simulink library "Garbage"  (R2023a)
settings: Solver=ode45  StartTime=0.0  StopTime=10.0  RelTol=1e-3  EnableMultiTasking=on
undefined symbols (not defined in the file or in its folder): c, ending_column_index, ending_row_index, framebuffer_16x1, framebuffer_2x2, framebuffer_4x4, framebuffer_8x8, HEIGHT, hot, POS_X, POS_Y, starting_column_index, starting_row_index, WIDTH
libraries: Memory_Blocks_Lib x459, simulink x235, simulink_extras x132, dspmtrx3 x116, Bit_Multiplexers_Lib x67, Bit_Demultiplexers_Lib x49, Byte_Multiplexers_Lib x40, Miscellaneous x38, Computer_Library x17, Decoders_Lib x14, commutil2 x12, Arithmetic_Operations_Lib x8
(part 10 of 26)

== system Garbage/Encoder_128_to_7 ==
  Select_127(1b) : Inport
  Select_126(1b) : Inport  Port=2
  Select_125(1b) : Inport  Port=3
  Select_124(1b) : Inport  Port=4
  Select_123(1b) : Inport  Port=5
  Select_122(1b) : Inport  Port=6
  Select_121(1b) : Inport  Port=7
  Select_120(1b) : Inport  Port=8
  Select_119(1b) : Inport  Port=9
  Select_118(1b) : Inport  Port=10
  Select_117(1b) : Inport  Port=11
  Select_116(1b) : Inport  Port=12
  Select_115(1b) : Inport  Port=13
  Select_114(1b) : Inport  Port=14
  Select_113(1b) : Inport  Port=15
  Select_112(1b) : Inport  Port=16
  Select_111(1b) : Inport  Port=17
  Select_110(1b) : Inport  Port=18
  Select_109(1b) : Inport  Port=19
  Select_108(1b) : Inport  Port=20
  Select_107(1b) : Inport  Port=21
  Select_106(1b) : Inport  Port=22
  Select_105(1b) : Inport  Port=23
  Select_104(1b) : Inport  Port=24
  Select_103(1b) : Inport  Port=25
  Select_102(1b) : Inport  Port=26
  Select_101(1b) : Inport  Port=27
  Select_100(1b) : Inport  Port=28
  Select_099(1b) : Inport  Port=29
  Select_098(1b) : Inport  Port=30
  Select_097(1b) : Inport  Port=31
  Select_096(1b) : Inport  Port=32
  Select_095(1b) : Inport  Port=33
  Select_094(1b) : Inport  Port=34
  Select_093(1b) : Inport  Port=35
  Select_092(1b) : Inport  Port=36
  Select_091(1b) : Inport  Port=37
  Select_090(1b) : Inport  Port=38
  Select_089(1b) : Inport  Port=39
  Select_088(1b) : Inport  Port=40
  Select_087(1b) : Inport  Port=41
  Select_086(1b) : Inport  Port=42
  Select_085(1b) : Inport  Port=43
  Select_084(1b) : Inport  Port=44
  Select_083(1b) : Inport  Port=45
  Select_082(1b) : Inport  Port=46
  Select_081(1b) : Inport  Port=47
  Select_080(1b) : Inport  Port=48
  Select_079(1b) : Inport  Port=49
  Select_078(1b) : Inport  Port=50
  Select_077(1b) : Inport  Port=51
  Select_076(1b) : Inport  Port=52
  Select_075(1b) : Inport  Port=53
  Select_074(1b) : Inport  Port=54
  Select_073(1b) : Inport  Port=55
  Select_072(1b) : Inport  Port=56
  Select_071(1b) : Inport  Port=57
  Select_070(1b) : Inport  Port=58
  Select_069(1b) : Inport  Port=59
  Select_068(1b) : Inport  Port=60
  Select_067(1b) : Inport  Port=61
  Select_066(1b) : Inport  Port=62
  Select_065(1b) : Inport  Port=63
  Select_064(1b) : Inport  Port=64
  Select_063(1b) : Inport  Port=65
  Select_062(1b) : Inport  Port=66
  Select_061(1b) : Inport  Port=67
  Select_060(1b) : Inport  Port=68
  Select_059(1b) : Inport  Port=69
  Select_058(1b) : Inport  Port=70
  Select_057(1b) : Inport  Port=71
  Select_056(1b) : Inport  Port=72
  Select_055(1b) : Inport  Port=73
  Select_054(1b) : Inport  Port=74
  Select_053(1b) : Inport  Port=75
  Select_052(1b) : Inport  Port=76
  Select_051(1b) : Inport  Port=77
  Select_050(1b) : Inport  Port=78
  Select_049(1b) : Inport  Port=79
  Select_048(1b) : Inport  Port=80
  Select_047(1b) : Inport  Port=81
  Select_046(1b) : Inport  Port=82
  Select_045(1b) : Inport  Port=83
  Select_044(1b) : Inport  Port=84
  Select_043(1b) : Inport  Port=85
  Select_042(1b) : Inport  Port=86
  Select_041(1b) : Inport  Port=87
  Select_040(1b) : Inport  Port=88
  Select_039(1b) : Inport  Port=89
  Select_038(1b) : Inport  Port=90
  Select_037(1b) : Inport  Port=91
  Select_036(1b) : Inport  Port=92
  Select_035(1b) : Inport  Port=93
  Select_034(1b) : Inport  Port=94
  Select_033(1b) : Inport  Port=95
  Select_032(1b) : Inport  Port=96
  Select_031(1b) : Inport  Port=97
  Select_030(1b) : Inport  Port=98
  Select_029(1b) : Inport  Port=99
  Select_028(1b) : Inport  Port=100
  Select_027(1b) : Inport  Port=101
  Select_026(1b) : Inport  Port=102
  Select_025(1b) : Inport  Port=103
  Select_024(1b) : Inport  Port=104
  Select_023(1b) : Inport  Port=105
  Select_022(1b) : Inport  Port=106
  Select_021(1b) : Inport  Port=107
  Select_020(1b) : Inport  Port=108
  Select_019(1b) : Inport  Port=109
  Select_018(1b) : Inport  Port=110
  Select_017(1b) : Inport  Port=111
  Select_016(1b) : Inport  Port=112
  Select_015(1b) : Inport  Port=113
  Select_014(1b) : Inport  Port=114
  Select_013(1b) : Inport  Port=115
  Select_012(1b) : Inport  Port=116
  Select_011(1b) : Inport  Port=117
  Select_010(1b) : Inport  Port=118
  Select_009(1b) : Inport  Port=119
  Select_008(1b) : Inport  Port=120
  Select_007(1b) : Inport  Port=121
  Select_006(1b) : Inport  Port=122
  Select_005(1b) : Inport  Port=123
  Select_004(1b) : Inport  Port=124
  Select_003(1b) : Inport  Port=125
  Select_002(1b) : Inport  Port=126
  Select_001(1b) : Inport  Port=127
  Select_000(1b) : Inport  Port=128
  Bus Creator : BusCreator  Inputs=8
  Logical Operator{1..7} (7 alike) : Logic  Operator=OR  Inputs=64  IconShape=distinctive  AllPortsSameDT=off  OutDataTypeStr=boolean
  Set1 : Constant  Value=0  OutDataTypeStr=boolean
  Terminator : Terminator
  Addr(1B) : Outport
  wires:
  Bus Creator/1 -> Addr(1B)/1
  Set1/1 -> Bus Creator/1
  Logical Operator7/1 -> Bus Creator/8
  Logical Operator5/1 -> Bus Creator/7
  Logical Operator4/1 -> Bus Creator/6
  Logical Operator3/1 -> Bus Creator/5
  Select_001(1b)/1 -> Logical Operator7/1
  Select_002(1b)/1 -> Logical Operator5/1
  Select_003(1b)/1 -> Logical Operator7/2, Logical Operator5/2
  Select_004(1b)/1 -> Logical Operator4/1
  Select_005(1b)/1 -> Logical Operator7/3, Logical Operator4/2
  Select_006(1b)/1 -> Logical Operator5/3, Logical Operator4/3
  Select_007(1b)/1 -> Logical Operator7/4, Logical Operator5/4, Logical Operator4/4
  Select_008(1b)/1 -> Logical Operator3/1
  Select_009(1b)/1 -> Logical Operator7/5, Logical Operator3/2
  Select_010(1b)/1 -> Logical Operator5/5, Logical Operator3/3
  Select_011(1b)/1 -> Logical Operator5/6, Logical Operator7/6, Logical Operator3/4
  Select_012(1b)/1 -> Logical Operator4/5, Logical Operator3/5
  Select_013(1b)/1 -> Logical Operator7/7, Logical Operator4/6, Logical Operator3/6
  Select_014(1b)/1 -> Logical Operator5/7, Logical Operator4/7, Logical Operator3/7
  Select_015(1b)/1 -> Logical Operator5/8, Logical Operator7/8, Logical Operator4/8, Logical Operator3/8
  Select_000(1b)/1 -> Terminator/1
  Logical Operator2/1 -> Bus Creator/4
  Select_016(1b)/1 -> Logical Operator2/1
  Select_017(1b)/1 -> Logical Operator7/9, Logical Operator2/2
  Select_018(1b)/1 -> Logical Operator5/9, Logical Operator2/3
  Select_019(1b)/1 -> Logical Operator7/10, Logical Operator5/10, Logical Operator2/4
  Select_020(1b)/1 -> Logical Operator4/9, Logical Operator2/5
  Select_021(1b)/1 -> Logical Operator7/11, Logical Operator4/10, Logical Operator2/6
  Select_022(1b)/1 -> Logical Operator5/11, Logical Operator4/11, Logical Operator2/7
  Select_023(1b)/1 -> Logical Operator7/12, Logical Operator5/12, Logical Operator4/12, Logical Operator2/8
  Select_024(1b)/1 -> Logical Operator3/9, Logical Operator2/9
  Select_025(1b)/1 -> Logical Operator7/13, Logical Operator3/10, Logical Operator2/10
  Select_026(1b)/1 -> Logical Operator2/11, Logical Operator5/13, Logical Operator3/11
  Select_027(1b)/1 -> Logical Operator7/14, Logical Operator5/14, Logical Operator3/12, Logical Operator2/12
  Select_028(1b)/1 -> Logical Operator3/13, Logical Operator4/13, Logical Operator2/13
  Select_029(1b)/1 -> Logical Operator7/15, Logical Operator4/14, Logical Operator3/14, Logical Operator2/14
  Select_030(1b)/1 -> Logical Operator5/15, Logical Operator4/15, Logical Operator3/15, Logical Operator2/15
  Select_031(1b)/1 -> Logical Operator7/16, Logical Operator5/16, Logical Operator4/16, Logical Operator3/16, Logical Operator2/16
  Logical Operator1/1 -> Bus Creator/3
  Select_032(1b)/1 -> Logical Operator1/1
  Select_033(1b)/1 -> Logical Operator7/17, Logical Operator1/2
  Select_034(1b)/1 -> Logical Operator5/17, Logical Operator1/3
  Select_035(1b)/1 -> Logical Operator7/18, Logical Operator5/18, Logical Operator1/4
  Select_036(1b)/1 -> Logical Operator1/5, Logical Operator4/17
  Select_037(1b)/1 -> Logical Operator7/19, Logical Operator4/18, Logical Operator1/6
  Select_039(1b)/1 -> Logical Operator7/20, Logical Operator5/20, Logical Operator4/20, Logical Operator1/8
  Select_038(1b)/1 -> Logical Operator5/19, Logical Operator4/19, Logical Operator1/7
  Select_040(1b)/1 -> Logical Operator3/17, Logical Operator1/9
  Select_041(1b)/1 -> Logical Operator3/18, Logical Operator7/21, Logical Operator1/10
  Select_043(1b)/1 -> Logical Operator7/22, Logical Operator5/22, Logical Operator3/20, Logical Operator1/12
  Select_044(1b)/1 -> Logical Operator4/21, Logical Operator3/21, Logical Operator1/13
  Select_045(1b)/1 -> Logical Operator7/23, Logical Operator4/22, Logical Operator3/22, Logical Operator1/14
  Select_046(1b)/1 -> Logical Operator5/23, Logical Operator4/23, Logical Operator3/23, Logical Operator1/15
  Select_047(1b)/1 -> Logical Operator7/24, Logical Operator5/24, Logical Operator4/24, Logical Operator3/24, Logical Operator1/16
  Select_048(1b)/1 -> Logical Operator2/17, Logical Operator1/17
  Select_049(1b)/1 -> Logical Operator7/25, Logical Operator2/18, Logical Operator1/18
  Select_050(1b)/1 -> Logical Operator5/25, Logical Operator2/19, Logical Operator1/19
  Select_051(1b)/1 -> Logical Operator7/26, Logical Operator5/26, Logical Operator2/20, Logical Operator1/20
  Select_052(1b)/1 -> Logical Operator4/25, Logical Operator2/21, Logical Operator1/21
  Select_053(1b)/1 -> Logical Operator7/27, Logical Operator4/26, Logical Operator2/22, Logical Operator1/22
  Select_055(1b)/1 -> Logical Operator7/28, Logical Operator5/28, Logical Operator4/28, Logical Operator2/24, Logical Operator1/24
  Select_056(1b)/1 -> Logical Operator3/25, Logical Operator2/25, Logical Operator1/25
  Select_057(1b)/1 -> Logical Operator7/29, Logical Operator3/26, Logical Operator2/26, Logical Operator1/26
  Select_058(1b)/1 -> Logical Operator5/29, Logical Operator3/27, Logical Operator2/27, Logical Operator1/27
  Select_059(1b)/1 -> Logical Operator7/30, Logical Operator5/30, Logical Operator3/28, Logical Operator2/28, Logical Operator1/28
  Select_061(1b)/1 -> Logical Operator7/31, Logical Operator4/30, Logical Operator3/30, Logical Operator2/30, Logical Operator1/30
  Select_062(1b)/1 -> Logical Operator5/31, Logical Operator4/31, Logical Operator3/31, Logical Operator2/31, Logical Operator1/31
  Select_063(1b)/1 -> Logical Operator7/32, Logical Operator5/32, Logical Operator4/32, Logical Operator3/32, Logical Operator2/32, Logical Operator1/32
  Select_042(1b)/1 -> Logical Operator5/21, Logical Operator3/19, Logical Operator1/11
  Select_054(1b)/1 -> Logical Operator5/27, Logical Operator4/27, Logical Operator2/23, Logical Operator1/23
  Select_060(1b)/1 -> Logical Operator4/29, Logical Operator3/29, Logical Operator2/29, Logical Operator1/29
  Logical Operator6/1 -> Bus Creator/2
  Select_065(1b)/1 -> Logical Operator7/33, Logical Operator6/2
  Select_066(1b)/1 -> Logical Operator5/33, Logical Operator6/3
  Select_067(1b)/1 -> Logical Operator7/34, Logical Operator5/34, Logical Operator6/4
  Select_068(1b)/1 -> Logical Operator4/33, Logical Operator6/5
  Select_069(1b)/1 -> Logical Operator7/35, Logical Operator4/34, Logical Operator6/6
  Select_070(1b)/1 -> Logical Operator5/35, Logical Operator4/35, Logical Operator6/7
  Select_071(1b)/1 -> Logical Operator7/36, Logical Operator5/36, Logical Operator4/36, Logical Operator6/8
  Select_072(1b)/1 -> Logical Operator3/33, Logical Operator6/9
  Select_073(1b)/1 -> Logical Operator7/37, Logical Operator3/34, Logical Operator6/10
  Select_074(1b)/1 -> Logical Operator5/37, Logical Operator3/35, Logical Operator6/11
  Select_075(1b)/1 -> Logical Operator7/38, Logical Operator5/38, Logical Operator3/36, Logical Operator6/12
  Select_076(1b)/1 -> Logical Operator4/37, Logical Operator3/37, Logical Operator6/13
  Select_077(1b)/1 -> Logical Operator7/39, Logical Operator4/38, Logical Operator3/38, Logical Operator6/14
  Select_078(1b)/1 -> Logical Operator5/39, Logical Operator4/39, Logical Operator3/39, Logical Operator6/15
  Select_079(1b)/1 -> Logical Operator4/40, Logical Operator5/40, Logical Operator7/40, Logical Operator3/40, Logical Operator6/16
  Select_064(1b)/1 -> Logical Operator6/1
  Select_080(1b)/1 -> Logical Operator2/33, Logical Operator6/17
  Select_081(1b)/1 -> Logical Operator7/41, Logical Operator2/34, Logical Operator6/18
  Select_082(1b)/1 -> Logical Operator2/35, Logical Operator5/41, Logical Operator6/19
  Select_083(1b)/1 -> Logical Operator7/42, Logical Operator5/42, Logical Operator2/36, Logical Operator6/20
  Select_084(1b)/1 -> Logical Operator4/41, Logical Operator2/37, Logical Operator6/21
  Select_085(1b)/1 -> Logical Operator6/22, Logical Operator7/43, Logical Operator4/42, Logical Operator2/38
  Select_086(1b)/1 -> Logical Operator5/43, Logical Operator4/43, Logical Operator2/39, Logical Operator6/23
  Select_087(1b)/1 -> Logical Operator4/44, Logical Operator5/44, Logical Operator7/44, Logical Operator2/40, Logical Operator6/24
  Select_088(1b)/1 -> Logical Operator3/41, Logical Operator2/41, Logical Operator6/25
  Select_089(1b)/1 -> Logical Operator7/45, Logical Operator3/42, Logical Operator2/42, Logical Operator6/26
  Select_090(1b)/1 -> Logical Operator5/45, Logical Operator3/43, Logical Operator2/43, Logical Operator6/27
  Select_091(1b)/1 -> Logical Operator7/46, Logical Operator5/46, Logical Operator3/44, Logical Operator2/44, Logical Operator6/28
  Select_092(1b)/1 -> Logical Operator4/45, Logical Operator3/45, Logical Operator2/45, Logical Operator6/29
  Select_093(1b)/1 -> Logical Operator2/46, Logical Operator3/46, Logical Operator7/47, Logical Operator4/46, Logical Operator6/30
  Select_094(1b)/1 -> Logical Operator5/47, Logical Operator4/47, Logical Operator3/47, Logical Operator2/47, Logical Operator6/31
  Select_095(1b)/1 -> Logical Operator7/48, Logical Operator5/48, Logical Operator4/48, Logical Operator3/48, Logical Operator2/48, Logical Operator6/32
  Select_096(1b)/1 -> Logical Operator1/33, Logical Operator6/33
  Select_098(1b)/1 -> Logical Operator5/49, Logical Operator1/35, Logical Operator6/35
  Select_099(1b)/1 -> Logical Operator7/50, Logical Operator5/50, Logical Operator1/36, Logical Operator6/36
  Select_100(1b)/1 -> Logical Operator4/49, Logical Operator1/37, Logical Operator6/37
  Select_101(1b)/1 -> Logical Operator7/51, Logical Operator4/50, Logical Operator1/38, Logical Operator6/38
  Select_103(1b)/1 -> Logical Operator7/52, Logical Operator5/52, Logical Operator4/52, Logical Operator1/40, Logical Operator6/40
  Select_102(1b)/1 -> Logical Operator5/51, Logical Operator4/51, Logical Operator1/39, Logical Operator6/39
  Select_104(1b)/1 -> Logical Operator3/49, Logical Operator1/41, Logical Operator6/41
  Select_105(1b)/1 -> Logical Operator7/53, Logical Operator3/50, Logical Operator1/42, Logical Operator6/42
  Select_107(1b)/1 -> Logical Operator7/54, Logical Operator5/54, Logical Operator3/52, Logical Operator1/44, Logical Operator6/44
  Select_108(1b)/1 -> Logical Operator4/53, Logical Operator3/53, Logical Operator1/45, Logical Operator6/45
  Select_109(1b)/1 -> Logical Operator6/46, Logical Operator4/54, Logical Operator7/55, Logical Operator3/54, Logical Operator1/46
  Select_110(1b)/1 -> Logical Operator3/55, Logical Operator4/55, Logical Operator5/55, Logical Operator1/47, Logical Operator6/47
  Select_111(1b)/1 -> Logical Operator7/56, Logical Operator5/56, Logical Operator4/56, Logical Operator3/56, Logical Operator1/48, Logical Operator6/48
  Select_112(1b)/1 -> Logical Operator1/49, Logical Operator2/49, Logical Operator6/49
  Select_114(1b)/1 -> Logical Operator5/57, Logical Operator2/51, Logical Operator1/51, Logical Operator6/51
  Select_115(1b)/1 -> Logical Operator7/58, Logical Operator5/58, Logical Operator2/52, Logical Operator1/52, Logical Operator6/52
  Select_116(1b)/1 -> Logical Operator4/57, Logical Operator2/53, Logical Operator1/53, Logical Operator6/53
  Select_117(1b)/1 -> Logical Operator4/58, Logical Operator7/59, Logical Operator2/54, Logical Operator1/54, Logical Operator6/54
  Select_119(1b)/1 -> Logical Operator7/60, Logical Operator5/60, Logical Operator4/60, Logical Operator2/56, Logical Operator1/56, Logical Operator6/56
  Select_121(1b)/1 -> Logical Operator1/58, Logical Operator2/58, Logical Operator3/58, Logical Operator7/61, Logical Operator6/58
  Select_122(1b)/1 -> Logical Operator5/61, Logical Operator3/59, Logical Operator2/59, Logical Operator1/59, Logical Operator6/59
  Select_123(1b)/1 -> Logical Operator7/62, Logical Operator5/62, Logical Operator3/60, Logical Operator2/60, Logical Operator1/60, Logical Operator6/60
  Select_125(1b)/1 -> Logical Operator4/62, Logical Operator7/63, Logical Operator3/62, Logical Operator2/62, Logical Operator1/62, Logical Operator6/62
  Select_126(1b)/1 -> Logical Operator6/63, Logical Operator1/63, Logical Operator5/63, Logical Operator4/63, Logical Operator3/63, Logical Operator2/63
  Select_127(1b)/1 -> Logical Operator5/64, Logical Operator7/64, Logical Operator4/64, Logical Operator3/64, Logical Operator2/64, Logical Operator1/64, Logical Operator6/64
  Select_106(1b)/1 -> Logical Operator6/43, Logical Operator1/43, Logical Operator5/53, Logical Operator3/51
  Select_118(1b)/1 -> Logical Operator5/59, Logical Operator4/59, Logical Operator2/55, Logical Operator1/55, Logical Operator6/55
  Select_124(1b)/1 -> Logical Operator3/61, Logical Operator4/61, Logical Operator2/61, Logical Operator1/61, Logical Operator6/61
  Select_120(1b)/1 -> Logical Operator2/57, Logical Operator3/57, Logical Operator1/57, Logical Operator6/57
  Select_113(1b)/1 -> Logical Operator7/57, Logical Operator2/50, Logical Operator1/50, Logical Operator6/50
  Select_097(1b)/1 -> Logical Operator1/34, Logical Operator7/49, Logical Operator6/34

== system Garbage/Graphic_Display_16x24 ==
  note: Individual words in video memory are interpreted as columns by the Matrix Concatenate block in the following way:
      | - Word with the highest address is interpreted as the left-most column.
      | - Word with the lowest address is interpreted as the right-most column.
      | - MSB bit is interpreted as the top pixel of a particular column.
      | - LSB bit is interpreted as the bottom pixel of a particular column.
      |
      | On screen we want to interpret the words as rows (to correspond to the memory layout) in the following way:
      | - Word with highest address represents top row.
      | - Word with lowest address represents bottom row.
      | - MSB bit represents left-most pixel.
      | - LSB bit represents right-most pixel.
      |
      | To achieve this the Permute Dimensions block is utilized.
  Video_In(8x2B) : Inport
  Video_In(16x2B) : Inport  Port=2
  Bus Selector1 : BusSelector  OutputSignals=signal1,signal2,signal3,signal4,signal5,signal6,signal7,signal8,signal9,signal10,signal11,signal12,signal13,signal14,signal15,signal16
  Bus Selector2 : BusSelector  OutputSignals=signal9,signal10,signal11,signal12,signal13,signal14,signal15,signal16
  Matrix Concatenate6 : Concatenate  NumInputs=24  Mode=Multidimensional array  ConcatenateDimension=2
  Matrix Viewer2 : dspobslib/Matrix Viewer  ImageParams=off  CMapStr=[0 0.3 0; 0 1 0]  AxisColorbar=on  AxisParams=off  AxisOrigin=Upper left corner  AxisTickMode=Auto  XTickRange=[0 1]  YTickRange=[0 1]  XLabel=X-axis  YLabel=Y-axis  ZLabel=Z-axis  FigPos=[1700 150 200 300]  AxisZoom=on
      settings: YMin=0  YMax=1
  Permute Dimensions : PermuteDimensions
  wires:
  Bus Selector2/3 -> Matrix Concatenate6/3  "<signal11>"
  Bus Selector1/12 -> Matrix Concatenate6/20  "<signal12>"
  Bus Selector1/16 -> Matrix Concatenate6/24  "<signal16>"
  Matrix Concatenate6/1 -> Permute Dimensions/1
  Bus Selector1/3 -> Matrix Concatenate6/11  "<signal3>"
  Bus Selector1/6 -> Matrix Concatenate6/14  "<signal6>"
  Bus Selector1/7 -> Matrix Concatenate6/15  "<signal7>"
  Bus Selector1/5 -> Matrix Concatenate6/13  "<signal5>"
  Bus Selector1/9 -> Matrix Concatenate6/17  "<signal9>"
  Permute Dimensions/1 -> Matrix Viewer2/1
  Video_In(8x2B)/1 -> Bus Selector2/1
  Bus Selector2/8 -> Matrix Concatenate6/8  "<signal16>"
  Bus Selector1/8 -> Matrix Concatenate6/16  "<signal8>"
  Bus Selector2/6 -> Matrix Concatenate6/6  "<signal14>"
  Bus Selector2/5 -> Matrix Concatenate6/5  "<signal13>"
  Bus Selector1/1 -> Matrix Concatenate6/9  "<signal1>"
  Bus Selector1/15 -> Matrix Concatenate6/23  "<signal15>"
  Bus Selector1/14 -> Matrix Concatenate6/22  "<signal14>"
  Bus Selector1/4 -> Matrix Concatenate6/12  "<signal4>"
  Bus Selector1/2 -> Matrix Concatenate6/10  "<signal2>"
  Bus Selector1/11 -> Matrix Concatenate6/19  "<signal11>"
  Bus Selector1/10 -> Matrix Concatenate6/18  "<signal10>"
  Bus Selector2/7 -> Matrix Concatenate6/7  "<signal15>"
  Video_In(16x2B)/1 -> Bus Selector1/1
  Bus Selector2/4 -> Matrix Concatenate6/4  "<signal12>"
  Bus Selector1/13 -> Matrix Concatenate6/21  "<signal13>"
  Bus Selector2/2 -> Matrix Concatenate6/2  "<signal10>"
  Bus Selector2/1 -> Matrix Concatenate6/1  "<signal9>"

== system Garbage/Keyboard1 ==
  note: Reset signal is ON when keyboard module is selected.
      | This signal resets already read character
      | when the character is copied from data bus.
  Reset(1b) : Inport
  CLK : Inport  Port=2
  ! : Constant  Value=0  OutDataTypeStr=boolean
  "\"" : Constant  Value=0  OutDataTypeStr=boolean
  "\"\\\"" : Constant  Value=0  OutDataTypeStr=boolean
  # : Constant  Value=0  OutDataTypeStr=boolean
  % : Constant  Value=0  OutDataTypeStr=boolean
  ( : Constant  Value=0  OutDataTypeStr=boolean
  ) : Constant  Value=0  OutDataTypeStr=boolean
  * : Constant  Value=0  OutDataTypeStr=boolean
  + : Constant  Value=0  OutDataTypeStr=boolean
  , : Constant  Value=0  OutDataTypeStr=boolean
  - : Constant  Value=0  OutDataTypeStr=boolean
  . : Constant  Value=0  OutDataTypeStr=boolean
  {0..9} (10 alike) : Constant  Value=0  OutDataTypeStr=boolean
  : : Constant  Value=0  OutDataTypeStr=boolean
  ; : Constant  Value=0  OutDataTypeStr=boolean
  < : Constant  Value=0  OutDataTypeStr=boolean
  = : Constant  Value=0  OutDataTypeStr=boolean
  > : Constant  Value=0  OutDataTypeStr=boolean
  "?" : Constant  Value=0  OutDataTypeStr=boolean
  A : Constant  Value=0  OutDataTypeStr=boolean
  Align{1..9} (9 alike) : Miscellaneous/Align8  SystemSampleTime=-1  VariantActivationTime=update diagram  Latency=0  AutoFrameSizeCalculation=off
  Align16 : Miscellaneous/Align16  SystemSampleTime=-1  VariantActivationTime=update diagram  Latency=0  AutoFrameSizeCalculation=off
  Alt : Constant  Value=0  OutDataTypeStr=boolean
  B : Constant  Value=0  OutDataTypeStr=boolean
  BckSpc : Constant  Value=0  OutDataTypeStr=boolean
  Bit to Integer Converter15 : simulink/Logic and Bit Operations/Bit to Integer Converter  nbits=16  bitOrder=MSB first  signedOutputValues=Unsigned  outDtype=uint16  outDtypeSigned=Inherit via internal rule
      settings: LibrarySourceBlock=|
      | commutil2/Bit to Integer
      | Converter
  Bit_{0..5} (6 alike) : simulink_extras/Flip Flops/J-K Flip-Flop  initial_condition=0
  Bitwise_NOT : Miscellaneous/Bitwise_NOT  SystemSampleTime=-1  VariantActivationTime=update diagram  Latency=0  AutoFrameSizeCalculation=off
  Bus Creator : BusCreator  Inputs=16
  Bus Creator1 : BusCreator  Inputs=8
  Bus Creator2 : BusCreator  Inputs=16
  Bus Selector1 : BusSelector  OutputSignals=signal11,signal12,signal13,signal14,signal15,signal16
  Bus Selector2 : BusSelector  OutputSignals=signal11,signal12,signal13,signal14,signal15,signal16
  Bus Selector4 : BusSelector  OutputSignals=signal1,signal2,signal3,signal4,signal5,signal6,signal7,signal8
  Byte_Orig : Memory_Blocks_Lib/Memory_Blocks_Lib/Byte_Orig  SystemSampleTime=-1  VariantActivationTime=update diagram  Latency=0  AutoFrameSizeCalculation=off
  C : Constant  Value=0  OutDataTypeStr=boolean
  D : Constant  Value=0  OutDataTypeStr=boolean
  Down : Constant  Value=0  OutDataTypeStr=boolean
  E : Constant  Value=0  OutDataTypeStr=boolean
  Enc_1 : Encoders_Lib/Enc_8  SystemSampleTime=-1  VariantActivationTime=update diagram  Latency=0  AutoFrameSizeCalculation=off
  Enc_8 : Encoders_Lib/Enc_8  SystemSampleTime=-1  VariantActivationTime=update diagram  Latency=0  AutoFrameSizeCalculation=off
  Enter : Constant  Value=0  OutDataTypeStr=boolean
  F : Constant  Value=0  OutDataTypeStr=boolean
  G : Constant  Value=0  OutDataTypeStr=boolean
  H : Constant  Value=0  OutDataTypeStr=boolean
  I : Constant  Value=0  OutDataTypeStr=boolean
  J : Constant  Value=0  OutDataTypeStr=boolean
  K : Constant  Value=0  OutDataTypeStr=boolean
  KeepOldValue (there is no reset and no button is pressed) : Logic  IconShape=distinctive  AllPortsSameDT=off  OutDataTypeStr=boolean
  L : Constant  Value=0  OutDataTypeStr=boolean
  Left : Constant  Value=0  OutDataTypeStr=boolean
  Logical Operator18 : Logic  Operator=NOT  IconShape=distinctive  AllPortsSameDT=off  OutDataTypeStr=boolean
  Logical Operator19 : Logic  Operator=NOT  IconShape=distinctive  AllPortsSameDT=off  OutDataTypeStr=boolean
  Logical Operator20 : Logic  Operator=NOT  IconShape=distinctive  AllPortsSameDT=off  OutDataTypeStr=boolean
  M : Constant  Value=0  OutDataTypeStr=boolean
  N : Constant  Value=0  OutDataTypeStr=boolean
  Name{20..27} (8 alike) : Miscellaneous/Name  SystemSampleTime=-1  VariantActivationTime=update diagram  Latency=0  AutoFrameSizeCalculation=off
  O : Constant  Value=0  OutDataTypeStr=boolean
  Or{1..16} (16 alike) : Logic  Operator=OR  Inputs=8  IconShape=distinctive  AllPortsSameDT=off  OutDataTypeStr=boolean
  P : Constant  Value=0  OutDataTypeStr=boolean
  Product : Product  Inputs=3
  Q : Constant  Value=0  OutDataTypeStr=boolean
  R : Constant  Value=0  OutDataTypeStr=boolean
  Reset (no button is pressed) : Logic  IconShape=distinctive  AllPortsSameDT=off  OutDataTypeStr=boolean
  Right : Constant  Value=0  OutDataTypeStr=boolean
  S : Constant  Value=0  OutDataTypeStr=boolean
  Set{1..2,4,6} (4 alike) : Constant  Value=0  OutDataTypeStr=boolean
  Set3 : Constant  Value=0  OutDataTypeStr=Bus: BUS_UINT16_T
  Shift : Constant  Value=0  OutDataTypeStr=boolean
  Slash : Constant  Value=0  OutDataTypeStr=boolean
  Space : Constant  Value=0  OutDataTypeStr=boolean
  Sum : Sum  Inputs=|++
  T : Constant  Value=0  OutDataTypeStr=boolean
  Terminator{2..7} (6 alike) : Terminator
  To Workspace1 : ToWorkspace  VariableName=keyboard  SampleTime=-1
      settings: MaxDataPoints=1  Save2DSignal=2-D array (concatenate along first dimension)
  U : Constant  Value=0  OutDataTypeStr=boolean
  Up : Constant  Value=0  OutDataTypeStr=boolean
  V : Constant  Value=0  OutDataTypeStr=boolean
  W : Constant  Value=0  OutDataTypeStr=boolean
  Word_Multiplexer_4_to_1 : Word_Multiplexers_Lib/Word_Multiplexers_Lib/Word_Multiplexer_4_to_1  SystemSampleTime=-1  VariantActivationTime=update diagram  Latency=0  AutoFrameSizeCalculation=off
  "Word_Multiplexer_4_to_1 " : Word_Multiplexers_Lib/Word_Multiplexers_Lib/Word_Multiplexer_4_to_1  SystemSampleTime=-1  VariantActivationTime=update diagram  Latency=0  AutoFrameSizeCalculation=off
  X : Constant  Value=0  OutDataTypeStr=boolean
  Y : Constant  Value=0  OutDataTypeStr=boolean
  Z : Constant  Value=0  OutDataTypeStr=boolean
  _ : Constant  Value=0  OutDataTypeStr=boolean
  isButtonPressed : Logic  Operator=OR  Inputs=6  IconShape=distinctive  AllPortsSameDT=off  OutDataTypeStr=boolean
  Button(2B) : Outport
  wires:
  Bus Creator/1 -> Bit to Integer Converter15/1, Button(2B)/1
  Bus Creator2/1 -> Word_Multiplexer_4_to_1/6
  Bit_5/2 -> Terminator2/1
  Bit_5/1 -> Bus Creator/11
  Bus Selector2/1 -> Bit_5/1  "<signal11>"
  Bit_4/2 -> Terminator3/1
  Bit_4/1 -> Bus Creator/12
  Bus Selector2/2 -> Bit_4/1  "<signal12>"
  Bit_3/2 -> Terminator4/1
  Bit_3/1 -> Bus Creator/13
  Bus Selector2/3 -> Bit_3/1  "<signal13>"
  Bit_2/2 -> Terminator5/1
  Bit_2/1 -> Bus Creator/14
  Bus Selector2/4 -> Bit_2/1  "<signal14>"
  Bit_1/2 -> Terminator6/1
  Bit_1/1 -> Bus Creator/15
  Bus Selector2/5 -> Bit_1/1  "<signal15>"
  Bit_0/2 -> Terminator7/1
  Bit_0/1 -> Bus Creator/16
  Reset(1b)/1 -> Logical Operator19/1, Reset (no button is pressed)/1  "Reset"
  Set1/1 -> Bus Creator/1, Bus Creator/2, Bus Creator/3, Bus Creator/4, Bus Creator/5, Bus Creator/6, Bus Creator/7, Bus Creator/8, Bus Creator/9, Bus Creator/10
  isButtonPressed/1 -> Logical Operator18/1, Logical Operator20/1
  Logical Operator18/1 -> KeepOldValue (there is no reset and no button is pressed)/2
  Logical Operator19/1 -> KeepOldValue (there is no reset and no button is pressed)/1
  Logical Operator20/1 -> Reset (no button is pressed)/2
  Bus Selector2/6 -> Bit_0/1  "<signal16>"
  Set3/1 -> "Word_Multiplexer_4_to_1 "/4, "Word_Multiplexer_4_to_1 "/3, "Word_Multiplexer_4_to_1 "/5, Word_Multiplexer_4_to_1/3, Word_Multiplexer_4_to_1/4, Word_Multiplexer_4_to_1/5
  Reset (no button is pressed)/1 -> Word_Multiplexer_4_to_1/2
  "Word_Multiplexer_4_to_1 "/1 -> Bus Selector1/1
  Bitwise_NOT/1 -> "Word_Multiplexer_4_to_1 "/6
  Bus Selector1/1 -> Bit_5/3  "<signal11>"
  Bus Selector1/2 -> Bit_4/3  "<signal12>"
  Bus Selector1/3 -> Bit_3/3  "<signal13>"
  Bus Selector1/4 -> Bit_2/3  "<signal14>"
  Bus Selector1/5 -> Bit_1/3  "<signal15>"
  Bus Selector1/6 -> Bit_0/3  "<signal16>"
  KeepOldValue (there is no reset and no button is pressed)/1 -> Word_Multiplexer_4_to_1/1, "Word_Multiplexer_4_to_1 "/2
  Word_Multiplexer_4_to_1/1 -> Bitwise_NOT/1, Bus Selector2/1
  CLK/1 -> Bit_0/2, Bit_1/2, Bit_2/2, Bit_3/2, Bit_4/2, Bit_5/2
  ? -> Bus Selector4/1
  Set6/1 -> Bus Creator2/2, Bus Creator2/3, Bus Creator2/4, Bus Creator2/5, Bus Creator2/6, Bus Creator2/8, Bus Creator2/7, Bus Creator2/1
  Name27/1 -> Bus Creator2/16
  Name26/1 -> Bus Creator2/15
  Name25/1 -> Bus Creator2/14
  Name24/1 -> Bus Creator2/13
  Name23/1 -> Bus Creator2/12
  Name22/1 -> Bus Creator2/11
  Name21/1 -> Bus Creator2/10
  Name20/1 -> Bus Creator2/9
  Bus Selector4/1 -> Name20/1  "<signal1>"
  Bus Selector4/2 -> Name21/1  "<signal2>"
  Bus Selector4/3 -> Name22/1, isButtonPressed/1  "<signal3>"
  Bus Selector4/4 -> Name23/1, isButtonPressed/2  "<signal4>"
  Bus Selector4/5 -> Name24/1, isButtonPressed/3  "<signal5>"
  Bus Selector4/6 -> Name25/1, isButtonPressed/4  "<signal6>"
  Bus Selector4/7 -> Name26/1, isButtonPressed/5  "<signal7>"
  Bus Selector4/8 -> Name27/1, isButtonPressed/6  "<signal8>"
  Set4/1 -> "Word_Multiplexer_4_to_1 "/1
  Bit to Integer Converter15/1 -> To Workspace1/1
  Or2/1 -> Align9/1
  Or3/1 -> Align9/2
  Or4/1 -> Align9/3
  Or5/1 -> Align9/4
  Or6/1 -> Align9/5
  Or7/1 -> Align9/6
  Or8/1 -> Align9/7
  Or9/1 -> Align9/8
  ,/1 -> Align8/1, Or10/1
  Space/1 -> Align8/2, Or10/2
  +/1 -> Align8/3, Or10/3
  -/1 -> Align8/4, Or10/4
  Up/1 -> Or10/5, Align8/5
  Down/1 -> Or10/6, Align8/6
  Left/1 -> Or10/7, Align8/7
  Right/1 -> Align8/8, Or10/8
  ./1 -> Or11/8, Align1/8
  ;/1 -> Or11/7, Align1/7
  :/1 -> Or11/6, Align1/6
  Enter/1 -> Or11/5, Align1/5
  _/1 -> Or11/4, Align1/4
  =/1 -> Or11/3, Align1/3
  */1 -> Or11/2, Align1/2
  "\"\\\""/1 -> Or11/1, Align1/1
  Or10/1 -> Enc_1/1
  Or11/1 -> Enc_1/2
  Slash/1 -> Or1/8, Align3/8
  BckSpc/1 -> Or1/7, Align3/7
  "\""/1 -> Or1/6, Align3/6
  %/1 -> Or1/5, Align3/5
  #/1 -> Or1/4, Align3/4
  >/1 -> Or1/3, Align3/3
  </1 -> Or1/2, Align3/2
  )/1 -> Or1/1, Align3/1
  Or1/1 -> Enc_1/3
  Or13/1 -> Enc_1/4
  Or12/1 -> Enc_1/5
  Or14/1 -> Enc_1/6
  Or15/1 -> Enc_1/7
  Or16/1 -> Enc_1/8
  (/1 -> Or13/1, Align2/1
  !/1 -> Or13/2, Align2/2
  "?"/1 -> Or13/3, Align2/3
  Z/1 -> Or13/4, Align2/4
  Y/1 -> Or13/5, Align2/5
  X/1 -> Or13/6, Align2/6
  W/1 -> Or13/7, Align2/7
  V/1 -> Or13/8, Align2/8
  Align8/8 -> Or2/8
  Align8/7 -> Or3/8
  Align8/6 -> Or4/8
  Align8/5 -> Or5/8
  Align8/4 -> Or6/8
  Align8/3 -> Or7/8
  Align8/2 -> Or8/8
  Align8/1 -> Or9/8
  Align1/8 -> Or2/7
  Align1/7 -> Or3/7
  Align1/6 -> Or4/7
  Align1/5 -> Or5/7
  Align1/4 -> Or6/7
  Align1/3 -> Or7/7
  Align1/2 -> Or8/7
  Align1/1 -> Or9/7
  Align3/8 -> Or2/6
  Align3/7 -> Or3/6
  Align3/6 -> Or4/6
  Align3/5 -> Or5/6
  Align3/4 -> Or6/6
  Align3/3 -> Or7/6
  Align3/2 -> Or8/6
  Align3/1 -> Or9/6
  Q/1 -> Or12/5, Align4/5
  R/1 -> Or12/4, Align4/4
  N/1 -> Or12/8, Align4/8
  T/1 -> Or12/2, Align4/2
  O/1 -> Or12/7, Align4/7
  S/1 -> Or12/3, Align4/3
  U/1 -> Or12/1, Align4/1
  P/1 -> Or12/6, Align4/6
  I/1 -> Or14/5, Align5/5
  J/1 -> Or14/4, Align5/4
  F/1 -> Or14/8, Align5/8
  L/1 -> Or14/2, Align5/2
  G/1 -> Or14/7, Align5/7
  K/1 -> Or14/3, Align5/3
  M/1 -> Or14/1, Align5/1
  H/1 -> Or14/6, Align5/6
  A/1 -> Or15/5, Align6/5
  B/1 -> Or15/4, Align6/4
  8/1 -> Or15/8, Align6/8
  D/1 -> Or15/2, Align6/2
  9/1 -> Or15/7, Align6/7
  C/1 -> Or15/3, Align6/3
  E/1 -> Or15/1, Align6/1
  0/1 -> Or15/6, Align6/6
  3/1 -> Or16/5, Align7/5
  4/1 -> Or16/4, Align7/4
  Set2/1 -> Or16/8, Align7/8
  6/1 -> Or16/2, Align7/2
  1/1 -> Or16/7, Align7/7
  5/1 -> Or16/3, Align7/3
  7/1 -> Or16/1, Align7/1
  2/1 -> Or16/6, Align7/6
  Align2/8 -> Or2/5
  Align4/8 -> Or2/4
  Align5/8 -> Or2/3
  Align6/8 -> Or2/2
  Align7/8 -> Or2/1
  Align2/7 -> Or3/5
  Align4/7 -> Or3/4
  Align5/7 -> Or3/3
  Align6/7 -> Or3/2
  Align7/7 -> Or3/1
  Align2/6 -> Or4/5
  Align4/6 -> Or4/4
  Align5/6 -> Or4/3
  Align6/6 -> Or4/2
  Align7/6 -> Or4/1
  Align2/5 -> Or5/5
  Align4/5 -> Or5/4
  Align5/5 -> Or5/3
  Align6/5 -> Or5/2
  Align7/5 -> Or5/1
  Align2/4 -> Or6/5
  Align4/4 -> Or6/4
  Align5/4 -> Or6/3
  Align6/4 -> Or6/2
  Align7/4 -> Or6/1
  Align2/3 -> Or7/5
  Align4/3 -> Or7/4
  Align5/3 -> Or7/3
  Align6/3 -> Or7/2
  Align7/3 -> Or7/1
  Align2/2 -> Or8/5
  Align4/2 -> Or8/4
  Align5/2 -> Or8/3
  Align6/2 -> Or8/2
  Align7/2 -> Or8/1
  Align2/1 -> Or9/5
  Align4/1 -> Or9/4
  Align5/1 -> Or9/3
  Align6/1 -> Or9/2
  Align7/1 -> Or9/1
  Align9/8 -> Enc_8/8
  Align9/7 -> Enc_8/7
  Align9/6 -> Enc_8/6
  Align9/5 -> Enc_8/5
  Align9/4 -> Enc_8/4
  Align9/3 -> Enc_8/3
  Align9/2 -> Enc_8/2
  Align9/1 -> Enc_8/1
  Enc_8/2 -> Bus Creator1/7  "b01"
  Enc_8/3 -> Bus Creator1/8  "b00"
  Enc_1/1 -> Bus Creator1/3  "b05"
  Enc_1/2 -> Bus Creator1/4  "b04"
  Enc_1/3 -> Bus Creator1/5  "b03"
  Enc_8/1 -> Bus Creator1/6  "b02"
  Alt/1 -> Bus Creator1/1  "b07"
  Shift/1 -> Bus Creator1/2  "b06"

== system Garbage/Log2  ==
  note: log2(n)
  In1 : Inport
  Action Port : ActionPort  ActionPortLabel=if(u1 ~= 0)
  Constant6 : Constant  Value=2
  Log5 : Math  Operator=log10
  Log6 : Math  Operator=log10
  Product6 : Product  Inputs=*/  OutDataTypeStr=double
  Out1 : Outport
  wires:
  In1/1 -> Log6/1
  Log5/1 -> Product6/2
  Log6/1 -> Product6/1
  Constant6/1 -> Log5/1
  Product6/1 -> Out1/1

== system Garbage/Log2 1 ==
  note: log2(n)
  In1 : Inport
  Action Port : ActionPort  ActionPortLabel=if(u1 ~= 0)
  Constant1 : Constant  Value=32  OutDataTypeStr=double
  Constant6 : Constant  Value=2
  Log5 : Math  Operator=log10
  Log6 : Math  Operator=log10
  Product6 : Product  Inputs=*/  OutDataTypeStr=double
  Sum4 : Sum  Inputs=|++  OutDataTypeStr=double
  Out1 : Outport
  wires:
  In1/1 -> Log6/1
  Log5/1 -> Product6/2
  Log6/1 -> Product6/1
  Constant6/1 -> Log5/1
  Product6/1 -> Sum4/1
  Constant1/1 -> Sum4/2
  Sum4/1 -> Out1/1

== system Garbage/MergeOutputs ==
  Data_1(2B) : Inport
  Data_0(2B) : Inport  Port=2
  Data_7(2B) : Inport  Port=3
  Data_6(2B) : Inport  Port=4
  Data_5(2B) : Inport  Port=5
  Data_4(2B) : Inport  Port=6
  Data_3(2B) : Inport  Port=7
  Data_2(2B) : Inport  Port=8
  Bus Creator1 : BusCreator  Inputs=16
  Bus Selector{1..8} (8 alike) : BusSelector  OutputSignals=b15,b14,b13,b12,b11,b10,b09,b08,b07,b06,b05,b04,b03,b02,b01,b00
  Logical Operator{1..112,128..143} (128 alike) : Logic  Commented=on  Inputs=1  IconShape=distinctive  AllPortsSameDT=off  OutDataTypeStr=boolean
  Merge{,1..15} (16 alike) : Merge  Inputs=8
  Data(2B) : Outport
  wires:
  Bus Creator1/1 -> Data(2B)/1
  Merge2/1 -> Bus Creator1/16  "b00"
  Merge/1 -> Bus Creator1/15  "b01"
  Merge15/1 -> Bus Creator1/14  "b02"
  Merge14/1 -> Bus Creator1/13  "b03"
  Merge13/1 -> Bus Creator1/11  "b05"
  Merge12/1 -> Bus Creator1/12  "b04"
  Merge11/1 -> Bus Creator1/10  "b06"
  Merge10/1 -> Bus Creator1/9  "b07"
  Merge8/1 -> Bus Creator1/8  "b08"
  Merge9/1 -> Bus Creator1/7  "b09"
  Merge7/1 -> Bus Creator1/6  "b10"
  Merge6/1 -> Bus Creator1/5  "b11"
  Merge4/1 -> Bus Creator1/4  "b12"
  Merge5/1 -> Bus Creator1/3  "b13"
  Merge3/1 -> Bus Creator1/2  "b14"
  Merge1/1 -> Bus Creator1/1  "b15"
  Data_0(2B)/1 -> Bus Selector8/1
  Bus Selector8/1 -> Merge1/8  "<b15>"
  Bus Selector8/2 -> Merge3/8  "<b14>"
  Bus Selector8/3 -> Merge5/8  "<b13>"
  Bus Selector8/4 -> Merge4/8  "<b12>"
  Bus Selector8/5 -> Merge6/8  "<b11>"
  Bus Selector8/6 -> Merge7/8  "<b10>"
  Bus Selector8/7 -> Merge9/8  "<b09>"
  Bus Selector8/8 -> Merge8/8  "<b08>"
  Bus Selector8/9 -> Merge10/8  "<b07>"
  Bus Selector8/10 -> Merge11/8  "<b06>"
  Bus Selector8/11 -> Merge13/8  "<b05>"
  Bus Selector8/12 -> Merge12/8  "<b04>"
  Bus Selector8/13 -> Merge14/8  "<b03>"
  Bus Selector8/14 -> Merge15/8  "<b02>"
  Bus Selector8/15 -> Merge/8  "<b01>"
  Bus Selector8/16 -> Merge2/8  "<b00>"
  Data_1(2B)/1 -> Bus Selector1/1
  Bus Selector1/14 -> Merge15/7  "<b02>"
  Bus Selector1/7 -> Merge9/7  "<b09>"
  Bus Selector1/4 -> Merge4/7  "<b12>"
  Bus Selector1/8 -> Merge8/7  "<b08>"
  Bus Selector1/16 -> Merge2/7  "<b00>"
  Bus Selector1/9 -> Merge10/7  "<b07>"
  Bus Selector1/13 -> Merge14/7  "<b03>"
  Bus Selector1/11 -> Merge13/7  "<b05>"
  Bus Selector1/15 -> Merge/7  "<b01>"
  Bus Selector1/12 -> Merge12/7  "<b04>"
  Bus Selector1/6 -> Merge7/7  "<b10>"
  Bus Selector1/2 -> Merge3/7  "<b14>"
  Bus Selector1/3 -> Merge5/7  "<b13>"
  Bus Selector1/10 -> Merge11/7  "<b06>"
  Bus Selector1/5 -> Merge6/7  "<b11>"
  Bus Selector1/1 -> Merge1/7  "<b15>"
  Data_2(2B)/1 -> Bus Selector2/1
  Data_3(2B)/1 -> Bus Selector3/1
  Data_7(2B)/1 -> Bus Selector7/1
  Data_5(2B)/1 -> Bus Selector5/1
  Data_4(2B)/1 -> Bus Selector4/1
  Data_6(2B)/1 -> Bus Selector6/1
  Bus Selector2/7 -> Merge9/6  "<b09>"
  Bus Selector2/9 -> Merge10/6  "<b07>"
  Bus Selector2/10 -> Merge11/6  "<b06>"
  Bus Selector2/11 -> Merge13/6  "<b05>"
  Bus Selector2/8 -> Merge8/6  "<b08>"
  Bus Selector2/5 -> Merge6/6  "<b11>"
  Bus Selector2/15 -> Merge/6  "<b01>"
  Bus Selector2/12 -> Merge12/6  "<b04>"
  Bus Selector2/1 -> Merge1/6  "<b15>"
  Bus Selector2/4 -> Merge4/6  "<b12>"
  Bus Selector2/14 -> Merge15/6  "<b02>"
  Bus Selector2/6 -> Merge7/6  "<b10>"
  Bus Selector2/3 -> Merge5/6  "<b13>"
  Bus Selector2/2 -> Merge3/6  "<b14>"
  Bus Selector2/13 -> Merge14/6  "<b03>"
  Bus Selector3/7 -> Merge9/5  "<b09>"
  Bus Selector3/9 -> Merge10/5  "<b07>"
  Bus Selector3/10 -> Merge11/5  "<b06>"
  Bus Selector3/11 -> Merge13/5  "<b05>"
  Bus Selector3/8 -> Merge8/5  "<b08>"
  Bus Selector3/5 -> Merge6/5  "<b11>"
  Bus Selector3/15 -> Merge/5  "<b01>"
  Bus Selector3/12 -> Merge12/5  "<b04>"
  Bus Selector3/1 -> Merge1/5  "<b15>"
  Bus Selector3/4 -> Merge4/5  "<b12>"
  Bus Selector3/14 -> Merge15/5  "<b02>"
  Bus Selector3/6 -> Merge7/5  "<b10>"
  Bus Selector3/3 -> Merge5/5  "<b13>"
  Bus Selector3/2 -> Merge3/5  "<b14>"
  Bus Selector3/13 -> Merge14/5  "<b03>"
  Bus Selector4/7 -> Merge9/4  "<b09>"
  Bus Selector4/9 -> Merge10/4  "<b07>"
  Bus Selector4/10 -> Merge11/4  "<b06>"
  Bus Selector4/11 -> Merge13/4  "<b05>"
  Bus Selector4/8 -> Merge8/4  "<b08>"
  Bus Selector4/5 -> Merge6/4  "<b11>"
  Bus Selector4/15 -> Merge/4  "<b01>"
  Bus Selector4/12 -> Merge12/4  "<b04>"
  Bus Selector4/1 -> Merge1/4  "<b15>"
  Bus Selector4/4 -> Merge4/4  "<b12>"
  Bus Selector4/14 -> Merge15/4  "<b02>"
  Bus Selector4/6 -> Merge7/4  "<b10>"
  Bus Selector4/3 -> Merge5/4  "<b13>"
  Bus Selector4/2 -> Merge3/4  "<b14>"
  Bus Selector4/13 -> Merge14/4  "<b03>"
  Bus Selector5/7 -> Merge9/3  "<b09>"
  Bus Selector5/9 -> Merge10/3  "<b07>"
  Bus Selector5/10 -> Merge11/3  "<b06>"
  Bus Selector5/11 -> Merge13/3  "<b05>"
  Bus Selector5/8 -> Merge8/3  "<b08>"
  Bus Selector5/5 -> Merge6/3  "<b11>"
  Bus Selector5/15 -> Merge/3  "<b01>"
  Bus Selector5/12 -> Merge12/3  "<b04>"
  Bus Selector5/1 -> Merge1/3  "<b15>"
  Bus Selector5/4 -> Merge4/3  "<b12>"
  Bus Selector5/14 -> Merge15/3  "<b02>"
  Bus Selector5/6 -> Merge7/3  "<b10>"
  Bus Selector5/3 -> Merge5/3  "<b13>"
  Bus Selector5/2 -> Merge3/3  "<b14>"
  Bus Selector5/13 -> Merge14/3  "<b03>"
  Bus Selector6/7 -> Merge9/2  "<b09>"
  Bus Selector6/9 -> Merge10/2  "<b07>"
  Bus Selector6/10 -> Merge11/2  "<b06>"
  Bus Selector6/11 -> Merge13/2  "<b05>"
  Bus Selector6/8 -> Merge8/2  "<b08>"
  Bus Selector6/5 -> Merge6/2  "<b11>"
  Bus Selector6/15 -> Merge/2  "<b01>"
  Bus Selector6/12 -> Merge12/2  "<b04>"
  Bus Selector6/1 -> Merge1/2  "<b15>"
  Bus Selector6/4 -> Merge4/2  "<b12>"
  Bus Selector6/14 -> Merge15/2  "<b02>"
  Bus Selector6/6 -> Merge7/2  "<b10>"
  Bus Selector6/3 -> Merge5/2  "<b13>"
  Bus Selector6/2 -> Merge3/2  "<b14>"
  Bus Selector6/13 -> Merge14/2  "<b03>"
  Bus Selector7/7 -> Merge9/1  "<b09>"
  Bus Selector7/9 -> Merge10/1  "<b07>"
  Bus Selector7/10 -> Merge11/1  "<b06>"
  Bus Selector7/11 -> Merge13/1  "<b05>"
  Bus Selector7/8 -> Merge8/1  "<b08>"
  Bus Selector7/5 -> Merge6/1  "<b11>"
  Bus Selector7/15 -> Merge/1  "<b01>"
  Bus Selector7/12 -> Merge12/1  "<b04>"
  Bus Selector7/1 -> Merge1/1  "<b15>"
  Bus Selector7/4 -> Merge4/1  "<b12>"
  Bus Selector7/14 -> Merge15/1  "<b02>"
  Bus Selector7/6 -> Merge7/1  "<b10>"
  Bus Selector7/3 -> Merge5/1  "<b13>"
  Bus Selector7/2 -> Merge3/1  "<b14>"
  Bus Selector7/13 -> Merge14/1  "<b03>"
  Bus Selector2/16 -> Merge2/6  "<b00>"
  Bus Selector3/16 -> Merge2/5  "<b00>"
  Bus Selector4/16 -> Merge2/4  "<b00>"
  Bus Selector5/16 -> Merge2/3  "<b00>"
  Bus Selector6/16 -> Merge2/2  "<b00>"
  Bus Selector7/16 -> Merge2/1  "<b00>"

== system Garbage/NOT_USED_Bit_Subtractor ==
  Data_In_A(1b) : Inport
  Data_In_B(1b) : Inport  Port=2
  Borrow_In(1b) : Inport  Port=3
  Logical Operator : Logic  IconShape=distinctive  AllPortsSameDT=off  OutDataTypeStr=boolean
  Logical Operator1 : Logic  IconShape=distinctive  AllPortsSameDT=off  OutDataTypeStr=boolean
  Logical Operator10 : Logic  Operator=NOT  Inputs=4  IconShape=distinctive  AllPortsSameDT=off  OutDataTypeStr=boolean
  Logical Operator11 : Logic  Operator=NOT  Inputs=4  IconShape=distinctive  AllPortsSameDT=off  OutDataTypeStr=boolean
  Logical Operator2 : Logic  IconShape=distinctive  AllPortsSameDT=off  OutDataTypeStr=boolean
  Logical Operator{3..6} (4 alike) : Logic  Inputs=3  IconShape=distinctive  AllPortsSameDT=off  OutDataTypeStr=boolean
  Logical Operator7 : Logic  Operator=OR  Inputs=4  IconShape=distinctive  AllPortsSameDT=off  OutDataTypeStr=boolean
  Logical Operator8 : Logic  Operator=OR  Inputs=3  IconShape=distinctive  AllPortsSameDT=off  OutDataTypeStr=boolean
  Logical Operator9 : Logic  Operator=NOT  Inputs=4  IconShape=distinctive  AllPortsSameDT=off  OutDataTypeStr=boolean
  Data_Out(1b) : Outport
  Borrow_Out(1b) : Outport  Port=2
  wires:
  Logical Operator11/1 -> Logical Operator4/3, Logical Operator5/3
  Logical Operator10/1 -> Logical Operator3/2, Logical Operator5/2
  Logical Operator9/1 -> Logical Operator3/1, Logical Operator4/1, Logical Operator/1, Logical Operator1/1
  Data_In_A(1b)/1 -> Logical Operator9/1, Logical Operator5/1, Logical Operator6/1
  Data_In_B(1b)/1 -> Logical Operator10/1, Logical Operator4/2, Logical Operator6/2, Logical Operator/2, Logical Operator2/1
  Borrow_In(1b)/1 -> Logical Operator11/1, Logical Operator3/3, Logical Operator6/3, Logical Operator1/2, Logical Operator2/2
  Logical Operator2/1 -> Logical Operator8/3
  Logical Operator1/1 -> Logical Operator8/2
  Logical Operator/1 -> Logical Operator8/1
  Logical Operator8/1 -> Borrow_Out(1b)/1
  Logical Operator7/1 -> Data_Out(1b)/1
  Logical Operator6/1 -> Logical Operator7/4
  Logical Operator5/1 -> Logical Operator7/3
  Logical Operator4/1 -> Logical Operator7/2
  Logical Operator3/1 -> Logical Operator7/1

== system Garbage/NOT_USED_Byte_Demultiplexer_1_to_2 ==
  Addr(1b) : Inport
  Data(1B) : Inport  Port=2
  1_to_2_Bit_Demultiplexer{,1..7} (8 alike) : Bit_Demultiplexers_Lib/Bit_Demultiplexers_Lib/Bit_Demultiplexer_1_to_2
  Bit to Integer Converter2 : commutil2/Bit to Integer Converter  nbits=8  bitOrder=MSB first  signedOutputValues=Unsigned  outDtype=uint8  outDtypeSigned=Inherit via internal rule
  Bit to Integer Converter3 : commutil2/Bit to Integer Converter  nbits=8  bitOrder=MSB first  signedOutputValues=Unsigned  outDtype=uint8  outDtypeSigned=Inherit via internal rule
  Byte_0 : Mux  Inputs=8
  Byte_1 : Mux  Inputs=8
  Demux1 : Demux  Outputs=8
  Integer to Bit Converter7 : commutil2/Integer to Bit Converter  nbits=8  signedInputValues=Unsigned  bitOrder=MSB first  outDtype=boolean
  Data_1(1B) : Outport
  Data_0(1B) : Outport  Port=2
  wires:
  Demux1/4 -> 1_to_2_Bit_Demultiplexer3/2
  Demux1/5 -> 1_to_2_Bit_Demultiplexer4/2
  Demux1/6 -> 1_to_2_Bit_Demultiplexer5/2
  Demux1/7 -> 1_to_2_Bit_Demultiplexer6/2
  Demux1/8 -> 1_to_2_Bit_Demultiplexer7/2
  Demux1/3 -> 1_to_2_Bit_Demultiplexer2/2
  Demux1/2 -> 1_to_2_Bit_Demultiplexer1/2
  Demux1/1 -> 1_to_2_Bit_Demultiplexer/2
  Byte_1/1 -> Bit to Integer Converter2/1
  Byte_0/1 -> Bit to Integer Converter3/1
  1_to_2_Bit_Demultiplexer7/2 -> Byte_0/8
  1_to_2_Bit_Demultiplexer7/1 -> Byte_1/8
  1_to_2_Bit_Demultiplexer6/2 -> Byte_0/7
  1_to_2_Bit_Demultiplexer6/1 -> Byte_1/7
  1_to_2_Bit_Demultiplexer5/2 -> Byte_0/6
  1_to_2_Bit_Demultiplexer5/1 -> Byte_1/6
  1_to_2_Bit_Demultiplexer4/2 -> Byte_0/5
  1_to_2_Bit_Demultiplexer4/1 -> Byte_1/5
  1_to_2_Bit_Demultiplexer3/2 -> Byte_0/4
  1_to_2_Bit_Demultiplexer3/1 -> Byte_1/4
  1_to_2_Bit_Demultiplexer2/2 -> Byte_0/3
  1_to_2_Bit_Demultiplexer2/1 -> Byte_1/3
  1_to_2_Bit_Demultiplexer1/2 -> Byte_0/2
  1_to_2_Bit_Demultiplexer1/1 -> Byte_1/2
  1_to_2_Bit_Demultiplexer/2 -> Byte_0/1
  1_to_2_Bit_Demultiplexer/1 -> Byte_1/1
  Integer to Bit Converter7/1 -> Demux1/1
  Data(1B)/1 -> Integer to Bit Converter7/1
  Addr(1b)/1 -> 1_to_2_Bit_Demultiplexer/1, 1_to_2_Bit_Demultiplexer7/1, 1_to_2_Bit_Demultiplexer6/1, 1_to_2_Bit_Demultiplexer5/1, 1_to_2_Bit_Demultiplexer4/1, 1_to_2_Bit_Demultiplexer3/1, 1_to_2_Bit_Demultiplexer2/1, 1_to_2_Bit_Demultiplexer1/1
  Bit to Integer Converter2/1 -> Data_1(1B)/1
  Bit to Integer Converter3/1 -> Data_0(1B)/1
